simulink model "fft_test"  (Simulink 7.4)
settings: Solver=ode45  StartTime=0.0  StopTime=5000  RelTol=1e-3
libraries: xbsIndex_r4 x2104, casper_library_misc x69, xbsReplacements_r4 x26, casper_library_delays x12, casper_library_reorder x2, casper_library_ffts_twiddle x1
(part 20 of 20)

== system fft_test/fft_xblock/fft_biplex/biplex_core/fft_stage_9/butterfly_direct/twiddle/coeff_gen ==
  rst : Inport
  Counter : xbsIndex_r4/Counter  cnt_type=Free Running  cnt_to=Inf  operation=Up  start_count=0  cnt_by_val=1  arith_type=Unsigned  n_bits=9  bin_pt=0  load_pin=off  rst=on  en=off  explicit_period=on  period=1  dbl_ovrd=off  use_behavioral_HDL=off  implementation=Fabric  block_type=counter
      settings: block_version=11.4
  ROM : xbsIndex_r4/ROM  depth=256  initVector=[1 0 0.707107543945312 -0.707107543945312 0.923873901367188 -0.3826904296875 0.3826904296875 -0.923873901367188 0.980789184570312 -0.195083618164062 0.555572509765625 -0.831466674804688 0.831466674804688 -0.555572509765625 0.195083618164062 -0.980789184570312 0.99517822265625 -0.0980224609375 0.6343994140625 -0.77301025390625 0.881927490234375 -0.471389770507812 0.290283203125 -0.956939697265625 0.956939697265625 -0.290283203125 0.471389770507812 -0.881927490234375 0.77301025390625 -0.6343994140625 0.0980224609375 -0.99517822265625 0.998794555664062 -0.049072265625 0.671554565429688 -0.740951538085938 0.90399169921875 -0.42755126953125 0.336883544921875 -0.941543579101562 0.97003173828125 -0.24298095703125 0.51409912109375 -0.85772705078125 0.803207397460938 -0.595703125 0.146728515625 -0.989181518554688 0.989181518554688 -0.146728515625 0.595703125 -0.803207397460938 0.85772705078125 -0.51409912109375 0.24298095703125 -0.97003173828125 0.941543579101562 -0.336883544921875 0.42755126953125 -0.90399169921875 0.740951538085938 -0.671554565429688 0.049072265625 -0.998794555664062 0.99969482421875 -0.0245361328125 0.689544677734375 -0.7242431640625 0.914215087890625 -0.405242919921875 0.359893798828125 -0.932998657226562 0.9757080078125 -0.219100952148438 0.535003662109375 -0.8448486328125 0.817581176757812 -0.5758056640625 0.17095947265625 -0.985275268554688 0.992477416992188 -0.122406005859375 0.615234375 -0.788345336914062 0.870086669921875 -0.492904663085938 0.266708374023438 -0.963775634765625 0.94952392578125 -0.313674926757812 0.449615478515625 -0.893218994140625 0.7572021484375 -0.653167724609375 0.0735626220703125 -0.997283935546875 0.997283935546875 -0.0735626220703125 0.653167724609375 -0.7572021484375 0.893218994140625 -0.449615478515625 0.313674926757812 -0.94952392578125 0.963775634765625 -0.266708374023438 0.492904663085938 -0.870086669921875 0.788345336914062 -0.615234375 0.122406005859375 -0.992477416992188 0.985275268554688 -0.17095947265625 0.5758056640625 -0.817581176757812 0.8448486328125 -0.535003662109375 0.219100952148438 -0.9757080078125 0.932998657226562 -0.359893798828125 0.405242919921875 -0.914215087890625 0.7242431640625 -0.689544677734375 0.0245361328125 -0.99969482421875 0.999923706054688 -0.01226806640625 0.698379516601562 -0.715728759765625 0.919113159179688 -0.393997192382812 0.371322631835938 -0.928512573242188 0.978317260742188 -0.207107543945312 0.545318603515625 -0.838226318359375 0.8245849609375 -0.56573486328125 0.18304443359375 -0.983108520507812 0.993911743164062 -0.1102294921875 0.624862670898438 -0.780731201171875 0.876068115234375 -0.482177734375 0.278518676757812 -0.960433959960938 0.95330810546875 -0.302001953125 0.460540771484375 -0.88763427734375 0.765167236328125 -0.643829345703125 0.0858001708984375 -0.996307373046875 0.998123168945312 -0.0613250732421875 0.66241455078125 -0.749130249023438 0.898681640625 -0.438613891601562 0.3253173828125 -0.945602416992188 0.96697998046875 -0.254867553710938 0.5035400390625 -0.863967895507812 0.79583740234375 -0.605514526367188 0.13458251953125 -0.99090576171875 0.9873046875 -0.158859252929688 0.585800170898438 -0.810455322265625 0.851348876953125 -0.52459716796875 0.231063842773438 -0.972946166992188 0.937332153320312 -0.348419189453125 0.416427612304688 -0.909164428710938 0.732650756835938 -0.68060302734375 0.03680419921875 -0.99932861328125 0.99932861328125 -0.03680419921875 0.68060302734375 -0.732650756835938 0.909164428710938 -0.416427612304688 0.348419189453125 -0.937332153320312 0.972946166992188 -0.231063842773438 0.52459716796875 -0.851348876953125 0.810455322265625 -0.585800170898438 0.158859252929688 -0.9873046875 0.99090576171875 -0.13458251953125 0.605514526367188 -0.79583740234375 0.863967895507812 -0.5035400390625 0.254867553710938 -0.96697998046875 0.945602416992188 -0.3253173828125 0.438613891601562 -0.898681640625 0.749130249023438 -0.66241455078125 0.0613250732421875 -0.998123168945312 0.996307373046875 -0.0858001708984375 ...(+540 chars)]  distributed_mem=Block RAM  rst=off  init_reg=0  en=off  latency=2  arith_type="Signed  (2's comp)"  n_bits=18  bin_pt=16  dbl_ovrd=off  optimize=Area  use_rpm=on  block_type=sprom
      settings: block_version=11.4
  ROM1 : xbsIndex_r4/ROM  depth=256  initVector=[0 -1 -0.707107543945312 -0.707107543945312 -0.3826904296875 -0.923873901367188 -0.923873901367188 -0.3826904296875 -0.195083618164062 -0.980789184570312 -0.831466674804688 -0.555572509765625 -0.555572509765625 -0.831466674804688 -0.980789184570312 -0.195083618164062 -0.0980224609375 -0.99517822265625 -0.77301025390625 -0.6343994140625 -0.471389770507812 -0.881927490234375 -0.956939697265625 -0.290283203125 -0.290283203125 -0.956939697265625 -0.881927490234375 -0.471389770507812 -0.6343994140625 -0.77301025390625 -0.99517822265625 -0.0980224609375 -0.049072265625 -0.998794555664062 -0.740951538085938 -0.671554565429688 -0.42755126953125 -0.90399169921875 -0.941543579101562 -0.336883544921875 -0.24298095703125 -0.97003173828125 -0.85772705078125 -0.51409912109375 -0.595703125 -0.803207397460938 -0.989181518554688 -0.146728515625 -0.146728515625 -0.989181518554688 -0.803207397460938 -0.595703125 -0.51409912109375 -0.85772705078125 -0.97003173828125 -0.24298095703125 -0.336883544921875 -0.941543579101562 -0.90399169921875 -0.42755126953125 -0.671554565429688 -0.740951538085938 -0.998794555664062 -0.049072265625 -0.0245361328125 -0.99969482421875 -0.7242431640625 -0.689544677734375 -0.405242919921875 -0.914215087890625 -0.932998657226562 -0.359893798828125 -0.219100952148438 -0.9757080078125 -0.8448486328125 -0.535003662109375 -0.5758056640625 -0.817581176757812 -0.985275268554688 -0.17095947265625 -0.122406005859375 -0.992477416992188 -0.788345336914062 -0.615234375 -0.492904663085938 -0.870086669921875 -0.963775634765625 -0.266708374023438 -0.313674926757812 -0.94952392578125 -0.893218994140625 -0.449615478515625 -0.653167724609375 -0.7572021484375 -0.997283935546875 -0.0735626220703125 -0.0735626220703125 -0.997283935546875 -0.7572021484375 -0.653167724609375 -0.449615478515625 -0.893218994140625 -0.94952392578125 -0.313674926757812 -0.266708374023438 -0.963775634765625 -0.870086669921875 -0.492904663085938 -0.615234375 -0.788345336914062 -0.992477416992188 -0.122406005859375 -0.17095947265625 -0.985275268554688 -0.817581176757812 -0.5758056640625 -0.535003662109375 -0.8448486328125 -0.9757080078125 -0.219100952148438 -0.359893798828125 -0.932998657226562 -0.914215087890625 -0.405242919921875 -0.689544677734375 -0.7242431640625 -0.99969482421875 -0.0245361328125 -0.01226806640625 -0.999923706054688 -0.715728759765625 -0.698379516601562 -0.393997192382812 -0.919113159179688 -0.928512573242188 -0.371322631835938 -0.207107543945312 -0.978317260742188 -0.838226318359375 -0.545318603515625 -0.56573486328125 -0.8245849609375 -0.983108520507812 -0.18304443359375 -0.1102294921875 -0.993911743164062 -0.780731201171875 -0.624862670898438 -0.482177734375 -0.876068115234375 -0.960433959960938 -0.278518676757812 -0.302001953125 -0.95330810546875 -0.88763427734375 -0.460540771484375 -0.643829345703125 -0.765167236328125 -0.996307373046875 -0.0858001708984375 -0.0613250732421875 -0.998123168945312 -0.749130249023438 -0.66241455078125 -0.438613891601562 -0.898681640625 -0.945602416992188 -0.3253173828125 -0.254867553710938 -0.96697998046875 -0.863967895507812 -0.5035400390625 -0.605514526367188 -0.79583740234375 -0.99090576171875 -0.13458251953125 -0.158859252929688 -0.9873046875 -0.810455322265625 -0.585800170898438 -0.52459716796875 -0.851348876953125 -0.972946166992188 -0.231063842773438 -0.348419189453125 -0.937332153320312 -0.909164428710938 -0.416427612304688 -0.68060302734375 -0.732650756835938 -0.99932861328125 -0.03680419921875 -0.03680419921875 -0.99932861328125 -0.732650756835938 -0.68060302734375 -0.416427612304688 -0.909164428710938 -0.937332153320312 -0.348419189453125 -0.231063842773438 -0.972946166992188 -0.851348876953125 -0.52459716796875 -0.585800170898438 -0.810455322265625 -0.9873046875 -0.158859252929688 -0.13458251953125 -0.99090576171875 -0.79583740234375 -0.605514526367188 -0.5035400390625 -0.863967895507812 -0.96697998046875 -0.254867553710938 -0.3253173828125 -0.945602416992188 -0.898681640625 -0.438613891601562 ...(+670 chars)]  distributed_mem=Block RAM  rst=off  init_reg=0  en=off  latency=2  arith_type="Signed  (2's comp)"  n_bits=18  bin_pt=16  dbl_ovrd=off  optimize=Area  use_rpm=on  block_type=sprom
      settings: block_version=11.4
  Slice : xbsIndex_r4/Slice  nbits=8  boolean_output=off  mode=Upper Bit Location + Width  bit1=0  base1=MSB of Input  bit0=0  base0=LSB of Input  dbl_ovrd=off  block_type=slice
  ri_to_c : casper_library_misc/ri_to_c  SystemSampleTime=-1
  w : Outport
  wires:
  Slice/1 -> ROM1/1, ROM/1
  ri_to_c/1 -> w/1
  ROM1/1 -> ri_to_c/2
  ROM/1 -> ri_to_c/1
  Counter/1 -> Slice/1
  rst/1 -> Counter/1

== system fft_test/fft_xblock/fft_biplex/biplex_core/fft_stage_9/butterfly_direct/twiddle/mults ==
  a1 : Inport
  b1 : Inport  Port=2
  a2 : Inport  Port=3
  b2 : Inport  Port=4
  a3 : Inport  Port=5
  b3 : Inport  Port=6
  a4 : Inport  Port=7
  b4 : Inport  Port=8
  mult_{1..4} (4 alike) : xbsIndex_r4/Mult  precision=Full  arith_type="Signed  (2's comp)"  n_bits=16  bin_pt=14  quantization="Round  (unbiased: +/- Inf)"  overflow=Saturate  en=off  latency=2  dbl_ovrd=off  use_behavioral_HDL=off  opt=Speed  use_embedded=on  optimum_pipeline=off  pipeline=on  use_rpm=on  placement_style=Triangular  block_type=mult
      settings: block_version=11.4
  c1 : Outport
  c2 : Outport  Port=2
  c3 : Outport  Port=3
  c4 : Outport  Port=4
  wires:
  mult_4/1 -> c4/1
  b4/1 -> mult_4/2
  a4/1 -> mult_4/1
  mult_3/1 -> c3/1
  b3/1 -> mult_3/2
  a3/1 -> mult_3/1
  mult_2/1 -> c2/1
  b2/1 -> mult_2/2
  a2/1 -> mult_2/1
  mult_1/1 -> c1/1
  b1/1 -> mult_1/2
  a1/1 -> mult_1/1
